simulink model "adm_pcie_9h7_dts2x_dual_4x100g_dsp_8b"  (R2019a)
settings: Solver=VariableStepAuto  StartTime=0.0  StopTime=10.0  RelTol=1e-3
undefined symbols (not defined in the file or in its folder): datetime, fft_stages, posixtime
libraries: xbsIndex_r4 x21832, casper_library_misc x264, casper_library_sources x16, casper_library_delays x14, xps_library x11, casper_library_reorder x10, casper_library_scopes x8
(part 5 of 225)

== system adm_pcie_9h7_dts2x_dual_4x100g_dsp_8b/pipeline0/reorder ==
  note: bram in big reorder manually replaced
      | with URAM via black box, because sysgen
      | is too stupid to let the US+HBM family
      | use URAM
  note: reorder 64 times for everything dynamically:
      | 64 time x 32 chan x 4 IF = 8192 long reorder
      | output:
      |
      | IF x chan [32] x time [64] x [chan [16] x pol[2] x 8+8-bit]
  note: time x chan [128] x IF[2] x pol [2] x chan [4] x 8+8-bit
  note: Interleave polarizations:
      |
      | time x chan [128] x [IF[2] x chan [4] x pol [2] x 8+8-bit]
  note: Interleave IFs:
      |
      | time x chan [32] x IF[4] x [chan[16] x pol [2] x 8+8-bit]
  note: time x chan reorder
      | in: IF x chan[32] x time[4] x time[16] x [chan[16] x pol[2] x 2Bytes]
      |
      | out: IF x chan [32] x time[4] x chan[16] x [time [16] x pol[2] x 8+8-bit]
  note: time x chan reorder
      |
      | out: IF x chan [32] x chan[16] x time[4] x [time [16] x pol[2] x 8+8-bit]
      |
      | =
      |
      | IF x chan[512] x time[4] x [time[16] x pol[2] x 8+8 bit]
  note: reorder 1 times for everything dynamically:
      | 1 time x 32 chan x 4 IF = 128 long reorder
      | output:
      |
      | IF x chan [32] x  [chan [16] x pol[2] x 8+8-bit]
  sync : Inport
  din : Inport  Port=2
  Constant1 : xbsIndex_r4/Constant  const=0  gui_display_data_type=Fixed-point  arith_type=Unsigned  n_bits=16*2*4  bin_pt=0  preci_type=Single  exp_width=8  frac_width=24  explicit_period=on  period=1  equ=P=C  opselect=C  inp2=PCIN>>17  opr=+  inp1=P  carry=CIN  dbl_ovrd=off  block_type=constant
  Constant2 : xbsIndex_r4/Constant  const=1  gui_display_data_type=Boolean  arith_type=Boolean  n_bits=16  bin_pt=14  preci_type=Single  exp_width=8  frac_width=24  explicit_period=on  period=1  equ=P=C  opselect=C  inp2=PCIN>>17  opr=+  inp1=P  carry=CIN  dbl_ovrd=off  block_type=constant
  Constant3 : xbsIndex_r4/Constant  const=1  gui_display_data_type=Boolean  arith_type=Boolean  n_bits=16  bin_pt=14  preci_type=Single  exp_width=8  frac_width=24  explicit_period=on  period=1  equ=P=C  opselect=C  inp2=PCIN>>17  opr=+  inp1=P  carry=CIN  dbl_ovrd=off  block_type=constant
  Constant5 : xbsIndex_r4/Constant  const=1  gui_display_data_type=Boolean  arith_type=Boolean  n_bits=16  bin_pt=14  preci_type=Single  exp_width=8  frac_width=24  explicit_period=on  period=1  equ=P=C  opselect=C  inp2=PCIN>>17  opr=+  inp1=P  carry=CIN  dbl_ovrd=off  block_type=constant
  Constant9 : xbsIndex_r4/Constant  const=0  gui_display_data_type=Fixed-point  arith_type=Unsigned  n_bits=16*2*4  bin_pt=0  preci_type=Single  exp_width=8  frac_width=24  explicit_period=on  period=1  equ=P=C  opselect=C  inp2=PCIN>>17  opr=+  inp1=P  carry=CIN  dbl_ovrd=off  block_type=constant
  bus_create1 : Subsystem  AncestorBlock=casper_library_flow_control/bus_create  mask(bus_create){inputNum=4}
      mask prompts: inputNum "Number of inputs:"
      mask init:
      | bus_create_init(gcb,...
      |     'inputNum', inputNum);
  bus_create2 : Subsystem  AncestorBlock=casper_library_flow_control/bus_create  mask(bus_create){inputNum=16}
      mask prompts: inputNum "Number of inputs:"
      mask init:
      | bus_create_init(gcb,...
      |     'inputNum', inputNum);
  bus_expand1 : Subsystem  AncestorBlock=casper_library_flow_control/bus_expand  mask(bus_expand){mode=divisions of equal size, outputNum=2, outputWidth=16*4*2, outputBinaryPt=0, outputArithmeticType=0, show_format=off, outputToWorkspace=off, variablePrefix=out, outputToModelAsWell=on}
      mask prompts: outputNum "Number of outputs:"; outputWidth "Output width:"; outputBinaryPt "Output binary point position:"; outputArithmeticType "Output arithmetic type (ufix=0, fix=1, bool=2):"; show_format "Print format string?"; outputToWorkspace "Output to workspace?"; variablePrefix "Variable name prefix:"; outputToModelAsWell "Output to model as well?"
      mask help:
      | Expands a 'bus' made using a bus_create or Xilinx
      | concat block.
      | The input will be divided into the specified number
      | of outputs with the specified arithmetic types.
      | Divisions are made from most significant bit.
      mask init: same as adm_pcie_9h7_dts2x_dual_4x100g_dsp_8b/pipeline0/autocorr/bus_expand
      settings: AttributesFormatString=2 outputs
      mask TypeOptions mode: divisions of equal size | divisions of arbitrary size
      mask Callback mode: bus_expand_callback();
      mask Callback outputWidth: bus_expand_callback();
      mask Callback outputToWorkspace: bus_expand_callback();
  bus_expand2 : Subsystem  AncestorBlock=casper_library_flow_control/bus_expand  mask(bus_expand){mode=divisions of equal size, outputNum=16, outputWidth=16*2, outputBinaryPt=0, outputArithmeticType=0, show_format=off, outputToWorkspace=off, variablePrefix=out, outputToModelAsWell=on}
      mask prompts: outputNum "Number of outputs:"; outputWidth "Output width:"; outputBinaryPt "Output binary point position:"; outputArithmeticType "Output arithmetic type (ufix=0, fix=1, bool=2):"; show_format "Print format string?"; outputToWorkspace "Output to workspace?"; variablePrefix "Variable name prefix:"; outputToModelAsWell "Output to model as well?"
      mask help:
      | Expands a 'bus' made using a bus_create or Xilinx
      | concat block.
      | The input will be divided into the specified number
      | of outputs with the specified arithmetic types.
      | Divisions are made from most significant bit.
      mask init: same as adm_pcie_9h7_dts2x_dual_4x100g_dsp_8b/pipeline0/autocorr/bus_expand
      settings: AttributesFormatString=16 outputs
      mask TypeOptions mode: divisions of equal size | divisions of arbitrary size
      mask Callback mode: bus_expand_callback();
      mask Callback outputWidth: bus_expand_callback();
      mask Callback outputToWorkspace: bus_expand_callback();
  interleave_pol0 : Subsystem
  interleave_pol1 : Subsystem
  reorder : Subsystem  AncestorBlock=casper_library_reorder/reorder  mask(reorder){map=reshape(reshape([0:64-1], 16, 4)', 1, 64), n_bits=512, n_inputs=1, bram_latency=3, map_latency=1, fanout_latency=0, double_buffer=0, bram_map=on, software_controlled=off}
      mask prompts: map "Output Order:"; n_bits "Data width in"; n_inputs "Number of inputs"; bram_latency "BRAM Latency"; map_latency "Map Latency"; fanout_latency "Fanout latency"; double_buffer "Double Buffer"; bram_map "Use BlockRAM for Map"; software_controlled "Use runtime software control"
      mask init:
      | reorder_init(gcb, ...
      |     'map', map, ...
      |     'n_bits', n_bits, ...
      |     'n_inputs', n_inputs, ...
      |     'bram_latency', bram_latency, ...
      |     'map_latency', map_latency, ...
      |     'fanout_latency', fanout_latency, ...
      |     'double_buffer', double_buffer, ...
      |     'bram_map', bram_map, ...
      |     'software_controlled', software_controlled);
      settings: AttributesFormatString=order=3
      mask Help: eval('xlWeb(''http://casper.berkeley.edu/wiki/Reorder'')')
  reorder1 : Subsystem  AncestorBlock=casper_library_reorder/reorder  mask(reorder){map=[128-1:-1:0], n_bits=512, n_inputs=1, bram_latency=3, map_latency=1, fanout_latency=0, double_buffer=1, bram_map=off, software_controlled=on}
      mask prompts: map "Output Order:"; n_bits "Data width in"; n_inputs "Number of inputs"; bram_latency "BRAM Latency"; map_latency "Map Latency"; fanout_latency "Fanout latency"; double_buffer "Double Buffer"; bram_map "Use BlockRAM for Map"; software_controlled "Use runtime software control"
      mask init: same as adm_pcie_9h7_dts2x_dual_4x100g_dsp_8b/pipeline0/reorder/reorder
      settings: AttributesFormatString=order=2
      mask Help: eval('xlWeb(''http://casper.berkeley.edu/wiki/Reorder'')')
  reorder3 : Subsystem  AncestorBlock=casper_library_reorder/reorder  mask(reorder){map=reshape(reshape([0:8192-1], 128, 64)', 1, 8192), n_bits=512, n_inputs=1, bram_latency=3, map_latency=2, fanout_latency=0, double_buffer=0, bram_map=on, software_controlled=off}
      mask prompts: map "Output Order:"; n_bits "Data width in"; n_inputs "Number of inputs"; bram_latency "BRAM Latency"; map_latency "Map Latency"; fanout_latency "Fanout latency"; double_buffer "Double Buffer"; bram_map "Use BlockRAM for Map"; software_controlled "Use runtime software control"
      mask init: same as adm_pcie_9h7_dts2x_dual_4x100g_dsp_8b/pipeline0/reorder/reorder
      settings: AttributesFormatString=order=13
      mask Help: eval('xlWeb(''http://casper.berkeley.edu/wiki/Reorder'')')
  square_transposer2 : Subsystem  AncestorBlock=casper_library_reorder/square_transposer  mask(square_transposer){n_inputs=4, async=off}
      mask prompts: n_inputs "Number of inputs (2^?):"; async "Aynchronus operation"
      mask init:
      | square_transposer_init(gcb,...
      |     'n_inputs', n_inputs, ...
      |     'async', async);
      settings: AttributesFormatString=n_inputs=4
      mask Help: eval('xlWeb(''http://casper.berkeley.edu/wiki/Square_transposer'')')
  square_transposer3 : Subsystem  AncestorBlock=casper_library_reorder/square_transposer  mask(square_transposer){n_inputs=2, async=off}
      mask prompts: n_inputs "Number of inputs (2^?):"; async "Aynchronus operation"
      mask init:
      | square_transposer_init(gcb,...
      |     'n_inputs', n_inputs, ...
      |     'async', async);
      settings: AttributesFormatString=n_inputs=2
      mask Help: eval('xlWeb(''http://casper.berkeley.edu/wiki/Square_transposer'')')
  sync_out : Outport
  dout : Outport  Port=2
  wires:
  bus_expand1/1(msb_out2) -> interleave_pol0/1(In1)
  bus_expand1/2(lsb_out1) -> interleave_pol1/1(In1)
  din/1 -> bus_expand1/1(bus_in)
  sync/1 -> square_transposer3/1(sync)
  interleave_pol0/1(Out1) -> square_transposer3/2(in0)
  Constant9/1 -> square_transposer3/5(in3)
  square_transposer3/2(out0) -> bus_create1/1(in1)
  square_transposer3/3(out1) -> bus_create1/2(in2)
  square_transposer3/4(out2) -> bus_create1/3(in3)
  square_transposer3/5(out3) -> bus_create1/4(in4)
  Constant5/1 -> reorder3/2(en)
  reorder3/3(dout0) -> bus_expand2/1(bus_in)
  reorder3/1(sync_out) -> square_transposer2/1(sync)
  bus_expand2/1(msb_out16) -> square_transposer2/2(in0)
  bus_expand2/2(out15) -> square_transposer2/3(in1)
  bus_expand2/3(out14) -> square_transposer2/4(in2)
  bus_expand2/4(out13) -> square_transposer2/5(in3)
  bus_expand2/5(out12) -> square_transposer2/6(in4)
  bus_expand2/6(out11) -> square_transposer2/7(in5)
  bus_expand2/7(out10) -> square_transposer2/8(in6)
  bus_expand2/8(out9) -> square_transposer2/9(in7)
  bus_expand2/9(out8) -> square_transposer2/10(in8)
  bus_expand2/10(out7) -> square_transposer2/11(in9)
  bus_expand2/11(out6) -> square_transposer2/12(in10)
  bus_expand2/12(out5) -> square_transposer2/13(in11)
  bus_expand2/13(out4) -> square_transposer2/14(in12)
  bus_expand2/14(out3) -> square_transposer2/15(in13)
  bus_expand2/15(out2) -> square_transposer2/16(in14)
  bus_expand2/16(lsb_out1) -> square_transposer2/17(in15)
  square_transposer2/2(out0) -> bus_create2/1(in1)
  square_transposer2/3(out1) -> bus_create2/2(in2)
  square_transposer2/4(out2) -> bus_create2/3(in3)
  square_transposer2/5(out3) -> bus_create2/4(in4)
  square_transposer2/6(out4) -> bus_create2/5(in5)
  square_transposer2/7(out5) -> bus_create2/6(in6)
  square_transposer2/8(out6) -> bus_create2/7(in7)
  square_transposer2/9(out7) -> bus_create2/8(in8)
  square_transposer2/10(out8) -> bus_create2/9(in9)
  square_transposer2/11(out9) -> bus_create2/10(in10)
  square_transposer2/12(out10) -> bus_create2/11(in11)
  square_transposer2/13(out11) -> bus_create2/12(in12)
  square_transposer2/14(out12) -> bus_create2/13(in13)
  square_transposer2/15(out13) -> bus_create2/14(in14)
  square_transposer2/16(out14) -> bus_create2/15(in15)
  square_transposer2/17(out15) -> bus_create2/16(in16)
  Constant1/1 -> square_transposer3/4(in2)
  interleave_pol1/1(Out1) -> square_transposer3/3(in1)
  bus_create2/1(bus_out) -> reorder/3(din0)
  square_transposer2/1(sync_out) -> reorder/1(sync)
  Constant2/1 -> reorder/2(en)
  reorder/1(sync_out) -> sync_out/1
  reorder/3(dout0) -> dout/1
  square_transposer3/1(sync_out) -> reorder1/1(sync)
  bus_create1/1(bus_out) -> reorder1/3(din0)
  Constant3/1 -> reorder1/2(en)
  reorder1/1(sync_out) -> reorder3/1(sync)
  reorder1/3(dout0) -> reorder3/3(din0)
